simulink model "ofdm_txrx_supermimo_coded"  (Simulink 7.1)
settings: Solver=VariableStepDiscrete  SolverMode=SingleTasking  StartTime=0.0  StopTime=2*simOnly_simLength  RelTol=1e-3
PostLoadFcn:
  | ofdm_rx_supermimo_init
InitFcn:
  | ofdm_rx_supermimo_init
StartFcn:
  | ofdm_rx_supermimo_init
values from neighbouring files (symbols this model uses):
  Action0_Config = 0* 31*2^18 + 0*2^24 + PHY_AUTORESPONSE_REQ_GOODHDR + PHY_AUTORESPONSE_REQ_GOODPKT + PHY_AUTORESPONSE_REQ_FLAGA    [ofdm_tx_supermimo_init.m]
  Action1_Config = 0* 62*2^18 + PHY_AUTORESPONSE_REQ_GOODHDR + PHY_AUTORESPONSE_REQ_GOODPKT    [ofdm_tx_supermimo_init.m]
  Action2_Config = 0    [ofdm_tx_supermimo_init.m]
  Action3_Config = 0    [ofdm_tx_supermimo_init.m]
  Action4_Config = 0    [ofdm_tx_supermimo_init.m]
  Action5_Config = 0    [ofdm_tx_supermimo_init.m]
  ActionID_AFTransmit = 31    [ofdm_tx_supermimo_init.m]
  ActionID_Disabled = 0    [ofdm_tx_supermimo_init.m]
  ActionID_SetFlagA = 62    [ofdm_tx_supermimo_init.m]
  ActionID_SetFlagB = 61    [ofdm_tx_supermimo_init.m]
  BER_RAM_init_size = 2048    [ofdm_tx_supermimo_init.m]
  channel_h = [1 0; 1 0] * 0.3    [ofdm_tx_supermimo_init.m]
  CPLength = 16    [ofdm_tx_supermimo_init.m]
  FEC_Config = fec_coding_en*1 + fec_soft_dec*2 + fec_qpsk_scl*2^4 + fec_16qam_scl*2^8    (= 4195)    [ofdm_tx_supermimo_init.m]
  longCorr_coef_i = [-4*real(LTS) 0]    [ofdm_rx_supermimo_init.m]
  longCorr_coef_q = [4*imag(LTS) 0]    [ofdm_rx_supermimo_init.m]
  Match0_Config = 0    [ofdm_tx_supermimo_init.m]
  Match1_Config = 0    [ofdm_tx_supermimo_init.m]
  Match2_Config = 0    [ofdm_tx_supermimo_init.m]
  Match3_Config = 0    [ofdm_tx_supermimo_init.m]
  Match4_Config = 0    [ofdm_tx_supermimo_init.m]
  Match5_Config = 0    [ofdm_tx_supermimo_init.m]
  Match6_Config = 0    [ofdm_tx_supermimo_init.m]
  Match7_Config = 0    [ofdm_tx_supermimo_init.m]
  max_CP_length = 16    [ofdm_tx_supermimo_init.m]
  max_num_baseRateSymbols = 31    [ofdm_tx_supermimo_init.m]
  max_num_subcarriers = 64    [ofdm_tx_supermimo_init.m]
  max_num_trainingSymbols = 15    [ofdm_tx_supermimo_init.m]
  max_numBytes = 16384    [ofdm_tx_supermimo_init.m]
  max_OFDM_symbols = 1023    [ofdm_tx_supermimo_init.m]
  modConstellation_qam16 = .75*[1 3 -1 -3]./3    [ofdm_tx_supermimo_init.m]
  modConstellation_qam64 = 0.875*[3 1 5 7 -3 -1 -5 -7]./7    [ofdm_tx_supermimo_init.m]
  modConstellation_qpsk = [1 -1]./sqrt(2)    [ofdm_tx_supermimo_init.m]
  numBaseRateSymbols = numBaseRateSymbols *2    [ofdm_tx_supermimo_init.m]
  numSubcarriers = 64    [ofdm_tx_supermimo_init.m]
  numTrainingSymbols = 2    [ofdm_tx_supermimo_init.m]
  pilot_indicies = 7 + ( (64-21) * 2^8) + ( (64-7) * 2^16) + (21 * 2^24)    (= 356068103)    [ofdm_tx_supermimo_init.m]
  pilotValues = hex2dec('A57D') + (2^16 * hex2dec('5A82'))    [ofdm_tx_supermimo_init.m]
  pktByteNums = numHeaderBytes +  				byteIndex_numPayloadBytes(1)*2^8 +  				byteIndex_numPayloadBytes(2)*2^16 +  				byteIndex_simpleDynModMasks * 2^24    [ofdm_rx_supermimo_init.m]
  PktDet_Delay = 58+32 +      Symbol_Timing_Offset*2^7 +      0*2^16 +      (117* 2^24)    (= 1962935642)    [ofdm_rx_supermimo_init.m]
  pktTiming_count_payloadStart = pktTiming_count_lsStart + pktTiming_ls_num*pktTiming_ls_length    [ofdm_rx_supermimo_init.m]
  preamble = preamble_scale*[shortsyms_10 longsyms_2]    [ofdm_tx_supermimo_init.m]
  preamble_ROM = preamble_I_ROM + preamble_Q_ROM*2^16    [ofdm_tx_supermimo_init.m]
  RAM_init_size = max_numBytes    (= 16384)    [ofdm_tx_supermimo_init.m]
  reg_AF_Tx_Blanking = (320+80) + ( (320+80+79)*2^16)    (= 31392144)    [ofdm_rx_supermimo_init.m]
  reg_AF_Tx_Scaling = 2944    [ofdm_rx_supermimo_init.m]
  reg_Interrupt_PktBuf_Control = reg_InterruptControl +  	1 * 2^16 +  	0 * 2^24    (= 65536)    [ofdm_rx_supermimo_init.m]
  reg_PilotCalcParams = (4 * 2^16) + (minPilotChanMag)    (= 262184.96)    [ofdm_rx_supermimo_init.m]
  regRx_chanEst_minMag = ((0.5)*2^15) + ((0.5 * 2^15)*2^16)    (= 1073758208)    [ofdm_rx_supermimo_init.m]
  regRx_coarseCalcCorrection = round((1.3e-3 - 1.2982e-3)*2^32)    [ofdm_rx_supermimo_init.m]
  regRx_pilotCalcCorrection = round(1.00115 * 2^31)    [ofdm_rx_supermimo_init.m]
  regRx_preCFOoptions = 0 * 2^0 +  	1 * 2^1 +  	0    (= 2)    [ofdm_rx_supermimo_init.m]
  rx_controlBits = 1 * 2  		 + 1 * 4  		 + 0 * 8  		 + 1 * 16 * rx_SISO_Mode  		 + 1 * 32  		 + 1 * 64  		 + 1 * 128  		 + 1 * 256  		 + 1 * 512  		 + 1 * 1024  		 + 1 * 4096  		 + 0 * 16384  		 + 0 * 32768  		 + 1 * 65536  		 + 0 * 131072  		 + 0 * 262144  		 + 1 * 524288  		 + 1 * 1048576 * tx_Alamouti_Mode  		 + 0 * 2097152           + 1 * 33554432  		 + 0 * 4194304  		 + 0 * 8388608  		 + 0 * 16777216  		 + 1 *  ...(+49 chars)    [ofdm_rx_supermimo_init.m]
  Rx_PktDet_LongCorr_Params = LongCorrCounterLoad + 0*2^8 + 0*2^16 + 255*2^24    (= 4278190325)    [ofdm_rx_supermimo_init.m]
  Rx_PktDet_LongCorrThresholds = 8000    [ofdm_rx_supermimo_init.m]
  rx_postEq_scaling = round(rxScaling*2^11) * (1 + 2^16)    [ofdm_rx_supermimo_init.m]
  RxDataScrambling_Seq = [40 198 78 63 82 173 102 245 48 111 172 115 147 230 216 93 72 65 62 2 205 242 122 90 128 83 105 97 73 10 5 252]    [ofdm_tx_supermimo_init.m]
  RxReg_FixedPktLen = 0    [ofdm_rx_supermimo_init.m]
  rxReg_pktDetCorr = 2^00 * (floor(0.7*128)) +  	2^08 * (300) +  	0    [ofdm_rx_supermimo_init.m]
  shortSymbol_time = shortSymbol_time(1:16).'    [ofdm_tx_supermimo_init.m]
  simOnly_numSamples = length(preamble)+( (numSubcarriers+CPLength)*(numTrainingSymbols + numBaseRateSymbols + numFullRateSymbols/2) )    [ofdm_tx_supermimo_init.m]
  train = [train train]    [ofdm_tx_supermimo_init.m]
  tx_controlBits = (antB_preambleShift * 2^4) + (2^1 * tx_Alamouti_Mode) +  	(2^0 * tx_SISO_Mode) + 4*0 + 8*1 + 0*256 + 512*0 + 1024*0 +  	0*hex2dec('1000') + 0*hex2dec('2000') + 0*hex2dec('4000') +  	1*hex2dec('8000') + 1*hex2dec('10000') + hex2dec('20000') +  	0*hex2dec('100000')    [ofdm_tx_supermimo_init.m]
  tx_delays = (50*2^0) + (0*2^8) + (30*2^12)    (= 122930)    [ofdm_tx_supermimo_init.m]
  tx_scaling = (3072) + (12288 * 2^16)    (= 805309440)    [ofdm_tx_supermimo_init.m]
  TxDataScrambling_Seq = [40 198 78 63 82 173 102 245 48 111 172 115 147 230 216 93 72 65 62 2 205 242 122 90 128 83 105 97 73 10 5 252]    [ofdm_tx_supermimo_init.m]
  txReg_symbolCounts = (2^8 *numBaseRateSymbols ) + numTrainingSymbols    [ofdm_tx_supermimo_init.m]
  TxRx_FFTScaling = (3 * 2^0) +  	(2 * 2^2) +  	(1 * 2^4) +  	(1 * 2^6) +  	(1 * 2^8) +  	(0 * 2^10)    (= 347)    [ofdm_tx_supermimo_init.m]
undefined symbols (not defined in the file or in its folder): AntA, AntB, BaseRate, Down, gcbh, numBuffs, numBytes, Up, xlBlockLoadCallback, xlGetSimulinkPeriod, xlipgetpartsetting
libraries: xbsIndex_r4 x6000, simulink x4, dspsigops x4, commchan3 x2, dspsrcs4 x2, commrflib2 x2
(part 5 of 63)

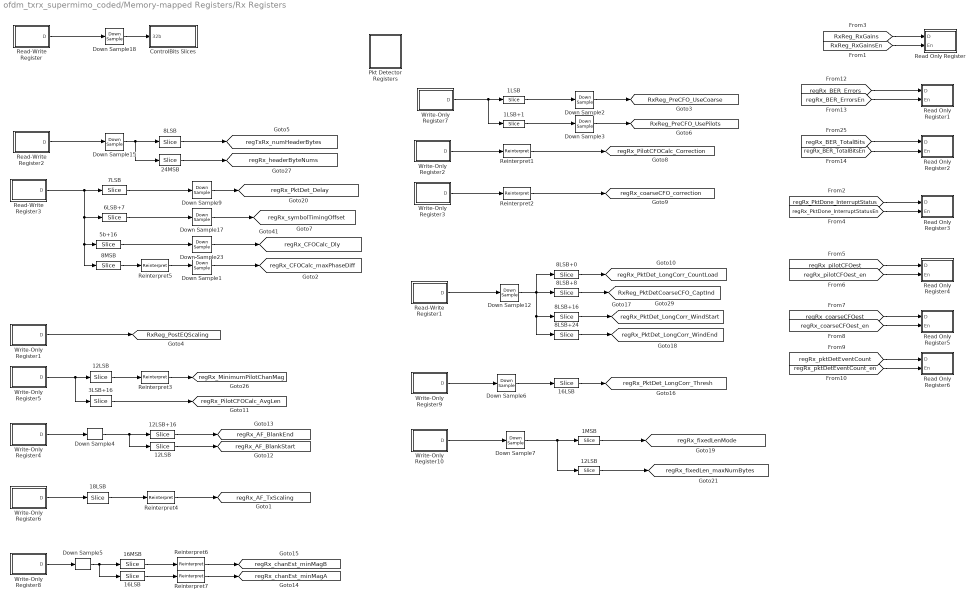
== system ofdm_txrx_supermimo_coded/Memory-mapped Registers/Rx Registers ==
  12LSB : xbsIndex_r4/Slice  nbits=12  boolean_output=off  mode=Lower Bit Location + Width  bit1=0  base1=MSB of Input  bit0=0  base0=LSB of Input  dbl_ovrd=off  block_type=slice
      settings: infoedit="Extracts a given range of bits from each input sample and presents it at the output.  The output type is ordinarily unsigned with binary point at zero, but can be Boolean when the slice is one bit wide.<P><P>Hardware notes: In hardware this block costs nothing."  block_version=VER_STRING_GOES_HERE
  "12LSB " : xbsIndex_r4/Slice  nbits=12  boolean_output=off  mode=Lower Bit Location + Width  bit1=0  base1=MSB of Input  bit0=0  base0=LSB of Input  dbl_ovrd=off  block_type=slice
      settings: infoedit="Extracts a given range of bits from each input sample and presents it at the output.  The output type is ordinarily unsigned with binary point at zero, but can be Boolean when the slice is one bit wide.<P><P>Hardware notes: In hardware this block costs nothing."  block_version=VER_STRING_GOES_HERE
  "12LSB  " : xbsIndex_r4/Slice  nbits=12  boolean_output=off  mode=Lower Bit Location + Width  bit1=0  base1=MSB of Input  bit0=0  base0=LSB of Input  dbl_ovrd=off  block_type=slice
      settings: infoedit="Extracts a given range of bits from each input sample and presents it at the output.  The output type is ordinarily unsigned with binary point at zero, but can be Boolean when the slice is one bit wide.<P><P>Hardware notes: In hardware this block costs nothing."  block_version=VER_STRING_GOES_HERE
  12LSB+16 : xbsIndex_r4/Slice  nbits=12  boolean_output=off  mode=Lower Bit Location + Width  bit1=0  base1=MSB of Input  bit0=16  base0=LSB of Input  dbl_ovrd=off  block_type=slice
      settings: infoedit="Extracts a given range of bits from each input sample and presents it at the output.  The output type is ordinarily unsigned with binary point at zero, but can be Boolean when the slice is one bit wide.<P><P>Hardware notes: In hardware this block costs nothing."  block_version=VER_STRING_GOES_HERE
  16LSB : xbsIndex_r4/Slice  nbits=16  boolean_output=off  mode=Lower Bit Location + Width  bit1=0  base1=MSB of Input  bit0=0  base0=LSB of Input  dbl_ovrd=off  block_type=slice
      settings: infoedit="Extracts a given range of bits from each input sample and presents it at the output.  The output type is ordinarily unsigned with binary point at zero, but can be Boolean when the slice is one bit wide.<P><P>Hardware notes: In hardware this block costs nothing."  block_version=VER_STRING_GOES_HERE
  "16LSB " : xbsIndex_r4/Slice  nbits=16  boolean_output=off  mode=Lower Bit Location + Width  bit1=0  base1=MSB of Input  bit0=0  base0=LSB of Input  dbl_ovrd=off  block_type=slice
      settings: infoedit="Extracts a given range of bits from each input sample and presents it at the output.  The output type is ordinarily unsigned with binary point at zero, but can be Boolean when the slice is one bit wide.<P><P>Hardware notes: In hardware this block costs nothing."  block_version=VER_STRING_GOES_HERE
  "16MSB  " : xbsIndex_r4/Slice  nbits=16  boolean_output=off  mode=Lower Bit Location + Width  bit1=0  base1=MSB of Input  bit0=16  base0=LSB of Input  dbl_ovrd=off  block_type=slice
      settings: infoedit="Extracts a given range of bits from each input sample and presents it at the output.  The output type is ordinarily unsigned with binary point at zero, but can be Boolean when the slice is one bit wide.<P><P>Hardware notes: In hardware this block costs nothing."  block_version=VER_STRING_GOES_HERE
  18LSB : xbsIndex_r4/Slice  nbits=18  boolean_output=off  mode=Lower Bit Location + Width  bit1=0  base1=MSB of Input  bit0=0  base0=LSB of Input  dbl_ovrd=off  block_type=slice
      settings: infoedit="Extracts a given range of bits from each input sample and presents it at the output.  The output type is ordinarily unsigned with binary point at zero, but can be Boolean when the slice is one bit wide.<P><P>Hardware notes: In hardware this block costs nothing."  block_version=VER_STRING_GOES_HERE
  "1LSB " : xbsIndex_r4/Slice  nbits=1  boolean_output=off  mode=Lower Bit Location + Width  bit1=0  base1=MSB of Input  bit0=0  base0=LSB of Input  dbl_ovrd=off  block_type=slice
      settings: infoedit="Extracts a given range of bits from each input sample and presents it at the output.  The output type is ordinarily unsigned with binary point at zero, but can be Boolean when the slice is one bit wide.<P><P>Hardware notes: In hardware this block costs nothing."  block_version=VER_STRING_GOES_HERE
  "1LSB+1 " : xbsIndex_r4/Slice  nbits=1  boolean_output=off  mode=Lower Bit Location + Width  bit1=0  base1=MSB of Input  bit0=1  base0=LSB of Input  dbl_ovrd=off  block_type=slice
      settings: infoedit="Extracts a given range of bits from each input sample and presents it at the output.  The output type is ordinarily unsigned with binary point at zero, but can be Boolean when the slice is one bit wide.<P><P>Hardware notes: In hardware this block costs nothing."  block_version=VER_STRING_GOES_HERE
  1MSB : xbsIndex_r4/Slice  nbits=1  boolean_output=off  mode=Upper Bit Location + Width  bit1=0  base1=MSB of Input  bit0=0  base0=LSB of Input  dbl_ovrd=off  block_type=slice
      settings: infoedit="Extracts a given range of bits from each input sample and presents it at the output.  The output type is ordinarily unsigned with binary point at zero, but can be Boolean when the slice is one bit wide.<P><P>Hardware notes: In hardware this block costs nothing."  block_version=VER_STRING_GOES_HERE
  24MSB : xbsIndex_r4/Slice  nbits=24  boolean_output=off  mode=Lower Bit Location + Width  bit1=0  base1=MSB of Input  bit0=8  base0=LSB of Input  dbl_ovrd=off  block_type=slice
      settings: infoedit="Extracts a given range of bits from each input sample and presents it at the output.  The output type is ordinarily unsigned with binary point at zero, but can be Boolean when the slice is one bit wide.<P><P>Hardware notes: In hardware this block costs nothing."  block_version=VER_STRING_GOES_HERE
  3LSB+16 : xbsIndex_r4/Slice  nbits=3  boolean_output=off  mode=Lower Bit Location + Width  bit1=0  base1=MSB of Input  bit0=16  base0=LSB of Input  dbl_ovrd=off  block_type=slice
      settings: infoedit="Extracts a given range of bits from each input sample and presents it at the output.  The output type is ordinarily unsigned with binary point at zero, but can be Boolean when the slice is one bit wide.<P><P>Hardware notes: In hardware this block costs nothing."  block_version=VER_STRING_GOES_HERE
  5b+16 : xbsIndex_r4/Slice  nbits=5  boolean_output=off  mode=Lower Bit Location + Width  bit1=0  base1=MSB of Input  bit0=16  base0=LSB of Input  dbl_ovrd=off  block_type=slice
      settings: infoedit="Extracts a given range of bits from each input sample and presents it at the output.  The output type is ordinarily unsigned with binary point at zero, but can be Boolean when the slice is one bit wide.<P><P>Hardware notes: In hardware this block costs nothing."  block_version=VER_STRING_GOES_HERE
  6LSB+7 : xbsIndex_r4/Slice  nbits=6  boolean_output=off  mode=Lower Bit Location + Width  bit1=0  base1=MSB of Input  bit0=7  base0=LSB of Input  dbl_ovrd=off  block_type=slice
      settings: infoedit="Extracts a given range of bits from each input sample and presents it at the output.  The output type is ordinarily unsigned with binary point at zero, but can be Boolean when the slice is one bit wide.<P><P>Hardware notes: In hardware this block costs nothing."  block_version=VER_STRING_GOES_HERE
  7LSB : xbsIndex_r4/Slice  nbits=7  boolean_output=off  mode=Lower Bit Location + Width  bit1=0  base1=MSB of Input  bit0=0  base0=LSB of Input  dbl_ovrd=off  block_type=slice
      settings: infoedit="Extracts a given range of bits from each input sample and presents it at the output.  The output type is ordinarily unsigned with binary point at zero, but can be Boolean when the slice is one bit wide.<P><P>Hardware notes: In hardware this block costs nothing."  block_version=VER_STRING_GOES_HERE
  "8LSB " : xbsIndex_r4/Slice  nbits=8  boolean_output=off  mode=Lower Bit Location + Width  bit1=0  base1=MSB of Input  bit0=0  base0=LSB of Input  dbl_ovrd=off  block_type=slice
      settings: infoedit="Extracts a given range of bits from each input sample and presents it at the output.  The output type is ordinarily unsigned with binary point at zero, but can be Boolean when the slice is one bit wide.<P><P>Hardware notes: In hardware this block costs nothing."  block_version=VER_STRING_GOES_HERE
  8LSB+0 : xbsIndex_r4/Slice  nbits=8  boolean_output=off  mode=Lower Bit Location + Width  bit1=0  base1=MSB of Input  bit0=0  base0=LSB of Input  dbl_ovrd=off  block_type=slice
      settings: infoedit="Extracts a given range of bits from each input sample and presents it at the output.  The output type is ordinarily unsigned with binary point at zero, but can be Boolean when the slice is one bit wide.<P><P>Hardware notes: In hardware this block costs nothing."  block_version=VER_STRING_GOES_HERE
  8LSB+16 : xbsIndex_r4/Slice  nbits=8  boolean_output=off  mode=Lower Bit Location + Width  bit1=0  base1=MSB of Input  bit0=16  base0=LSB of Input  dbl_ovrd=off  block_type=slice
      settings: infoedit="Extracts a given range of bits from each input sample and presents it at the output.  The output type is ordinarily unsigned with binary point at zero, but can be Boolean when the slice is one bit wide.<P><P>Hardware notes: In hardware this block costs nothing."  block_version=VER_STRING_GOES_HERE
  8LSB+24 : xbsIndex_r4/Slice  nbits=8  boolean_output=off  mode=Lower Bit Location + Width  bit1=0  base1=MSB of Input  bit0=24  base0=LSB of Input  dbl_ovrd=off  block_type=slice
      settings: infoedit="Extracts a given range of bits from each input sample and presents it at the output.  The output type is ordinarily unsigned with binary point at zero, but can be Boolean when the slice is one bit wide.<P><P>Hardware notes: In hardware this block costs nothing."  block_version=VER_STRING_GOES_HERE
  8LSB+8 : xbsIndex_r4/Slice  nbits=8  boolean_output=off  mode=Lower Bit Location + Width  bit1=0  base1=MSB of Input  bit0=8  base0=LSB of Input  dbl_ovrd=off  block_type=slice
      settings: infoedit="Extracts a given range of bits from each input sample and presents it at the output.  The output type is ordinarily unsigned with binary point at zero, but can be Boolean when the slice is one bit wide.<P><P>Hardware notes: In hardware this block costs nothing."  block_version=VER_STRING_GOES_HERE
  8MSB : xbsIndex_r4/Slice  nbits=8  boolean_output=off  mode=Lower Bit Location + Width  bit1=0  base1=MSB of Input  bit0=24  base0=LSB of Input  dbl_ovrd=off  block_type=slice
      settings: infoedit="Extracts a given range of bits from each input sample and presents it at the output.  The output type is ordinarily unsigned with binary point at zero, but can be Boolean when the slice is one bit wide.<P><P>Hardware notes: In hardware this block costs nothing."  block_version=VER_STRING_GOES_HERE
  ControlBits Slices : Subsystem
  Down Sample{1..4,6,9,12,23} (8 alike) : xbsIndex_r4/Down Sample  sample_ratio=4  sample_phase="Last Value of Frame  (most efficient)"  en=off  latency=1  dbl_ovrd=off  block_type=dsamp
      settings: block_version=VER_STRING_GOES_HERE
  Down Sample{5,7,15,17..18} (5 alike) : xbsIndex_r4/Down Sample  sample_ratio=2  sample_phase="Last Value of Frame  (most efficient)"  en=off  latency=1  dbl_ovrd=off  block_type=dsamp
      settings: block_version=VER_STRING_GOES_HERE
  Pkt Detector Registers : Subsystem
  Read Only Register : Subsystem  AncestorBlock=|  mask{regName='Rx_Gains'}
      | WARP_Blockset/Register Wrappers
      | (Sysgen 10.1+)/Read Only Register
      mask prompts: regName "Register Name"
      mask help:
      | This block is a wrapper for System Generator's To Register shared memory block. This register is designed for use with WARP's sysgen2opb tool.
      |
      | Note: The Regisger Name paramter below must be unique for every instance of Read-Write and Read-Only registers in your system.
      mask init:
      | regBlock = find_system(gcb, 'LookUnderMasks', 'all', 'FollowLinks', 'on',   'block_type', 'toreg');
      | 
      | if(length(regBlock) > 0)
      | 
      |     %Set the shared memory name
      |     set_param(regBlock{1}, 'shared_memory_name', regName);
      | 
      | 	set_param(regBlock{1}, 'explicit_data_type', 'on')
      |     set_param(regBlock{1}, 'arith_type', 'unsigned');
      | 	set_param(regBlock{1}, 'ownership', 'Locally owned and initialized')
      | 
      | 	set_param(regBlock{1}, 'n_bits', '32');
      | 	set_param(regBlock{1}, 'bin_pt', '0');
      | 	set_param(regBlock{1}, 'init', '0');
      | end
      settings: AttributesFormatString=RO Reg: %<regName>
  Read Only Register1 : Subsystem  AncestorBlock=|  mask{regName='Rx_BER_Errors'}
      | WARP_Blockset/Register Wrappers
      | (Sysgen 10.1+)/Read Only Register
      mask prompts: regName "Register Name"
      mask help:
      | This block is a wrapper for System Generator's To Register shared memory block. This register is designed for use with WARP's sysgen2opb tool.
      |
      | Note: The Regisger Name paramter below must be unique for every instance of Read-Write and Read-Only registers in your system.
      mask init: same as ofdm_txrx_supermimo_coded/Memory-mapped Registers/Rx Registers/Read Only Register
      settings: AttributesFormatString=RO Reg: %<regName>
  Read Only Register2 : Subsystem  AncestorBlock=|  mask{regName='Rx_BER_TotalBits'}
      | WARP_Blockset/Register Wrappers
      | (Sysgen 10.1+)/Read Only Register
      mask prompts: regName "Register Name"
      mask help:
      | This block is a wrapper for System Generator's To Register shared memory block. This register is designed for use with WARP's sysgen2opb tool.
      |
      | Note: The Regisger Name paramter below must be unique for every instance of Read-Write and Read-Only registers in your system.
      mask init: same as ofdm_txrx_supermimo_coded/Memory-mapped Registers/Rx Registers/Read Only Register
      settings: AttributesFormatString=RO Reg: %<regName>
  Read Only Register3 : Subsystem  AncestorBlock=|  mask{regName='Rx_pktDone_interruptStatus'}
      | WARP_Blockset/Register Wrappers
      | (Sysgen 10.1+)/Read Only Register
      mask prompts: regName "Register Name"
      mask help:
      | This block is a wrapper for System Generator's To Register shared memory block. This register is designed for use with WARP's sysgen2opb tool.
      |
      | Note: The Regisger Name paramter below must be unique for every instance of Read-Write and Read-Only registers in your system.
      mask init: same as ofdm_txrx_supermimo_coded/Memory-mapped Registers/Rx Registers/Read Only Register
      settings: AttributesFormatString=RO Reg: %<regName>
  Read Only Register4 : Subsystem  AncestorBlock=|  mask{regName='Rx_pilotCFOest'}
      | WARP_Blockset/Register Wrappers
      | (Sysgen 10.1+)/Read Only Register
      mask prompts: regName "Register Name"
      mask help:
      | This block is a wrapper for System Generator's To Register shared memory block. This register is designed for use with WARP's sysgen2opb tool.
      |
      | Note: The Regisger Name paramter below must be unique for every instance of Read-Write and Read-Only registers in your system.
      mask init: same as ofdm_txrx_supermimo_coded/Memory-mapped Registers/Rx Registers/Read Only Register
      settings: AttributesFormatString=RO Reg: %<regName>
  Read Only Register5 : Subsystem  AncestorBlock=|  mask{regName='Rx_coarseCFOest'}
      | WARP_Blockset/Register Wrappers
      | (Sysgen 10.1+)/Read Only Register
      mask prompts: regName "Register Name"
      mask help:
      | This block is a wrapper for System Generator's To Register shared memory block. This register is designed for use with WARP's sysgen2opb tool.
      |
      | Note: The Regisger Name paramter below must be unique for every instance of Read-Write and Read-Only registers in your system.
      mask init: same as ofdm_txrx_supermimo_coded/Memory-mapped Registers/Rx Registers/Read Only Register
      settings: AttributesFormatString=RO Reg: %<regName>
  Read Only Register6 : Subsystem  AncestorBlock=|  mask{regName='Rx_pktDetEventCount'}
      | WARP_Blockset/Register Wrappers
      | (Sysgen 10.1+)/Read Only Register
      mask prompts: regName "Register Name"
      mask help:
      | This block is a wrapper for System Generator's To Register shared memory block. This register is designed for use with WARP's sysgen2opb tool.
      |
      | Note: The Regisger Name paramter below must be unique for every instance of Read-Write and Read-Only registers in your system.
      mask init: same as ofdm_txrx_supermimo_coded/Memory-mapped Registers/Rx Registers/Read Only Register
      settings: AttributesFormatString=RO Reg: %<regName>
  Read-Write Register : Subsystem  AncestorBlock=|  mask{regName='Rx_ControlBits', out_dataType=Unsigned, out_numBits=32, out_binaryPt=0, initValue=rx_controlBits, sampPeriod=1}
      | WARP_Blockset/Register Wrappers
      | (Sysgen 10.1+)/Read-Write Register
      mask prompts: regName "Register Name"; out_dataType "Output Type"; out_numBits "Output Num Bits"; out_binaryPt "Output Binary Point"; initValue "Initial Value"; sampPeriod "Sample Period"
      mask help:
      | This block is a wrapper for two registers from the System Generator Shared Memory blockset:
      | a) A "From Register" block, which provides a write-only register for the host processor
      | b) A "To Register" block, which provides a read-only register for the host processor
      |
      | Note: The Regisger Name paramter below must be unique for every instance of shared-memory registers in your system. System Generator will throw an error if duplicate names are found.
      mask init: same as ofdm_txrx_supermimo_coded/Memory-mapped Registers/AutoReply Registers/Read-Write Register1
      settings: AttributesFormatString=RW Reg: %<regName>
      mask StyleString: edit,popup(Unsigned|Signed  (2's comp)),edit,edit,edit,edit
  Read-Write Register1 : Subsystem  AncestorBlock=|  mask{regName='Rx_PktDet_LongCorr_Params', out_dataType=Unsigned, out_numBits=32, out_binaryPt=0, initValue=Rx_PktDet_LongCorr_Params, sampPeriod=1}
      | WARP_Blockset/Register Wrappers
      | (Sysgen 10.1+)/Read-Write Register
      mask prompts: regName "Register Name"; out_dataType "Output Type"; out_numBits "Output Num Bits"; out_binaryPt "Output Binary Point"; initValue "Initial Value"; sampPeriod "Sample Period"
      mask help:
      | This block is a wrapper for two registers from the System Generator Shared Memory blockset:
      | a) A "From Register" block, which provides a write-only register for the host processor
      | b) A "To Register" block, which provides a read-only register for the host processor
      |
      | Note: The Regisger Name paramter below must be unique for every instance of shared-memory registers in your system. System Generator will throw an error if duplicate names are found.
      mask init: same as ofdm_txrx_supermimo_coded/Memory-mapped Registers/AutoReply Registers/Read-Write Register1
      settings: AttributesFormatString=RW Reg: %<regName>
      mask StyleString: edit,popup(Unsigned|Signed  (2's comp)),edit,edit,edit,edit
  Read-Write Register2 : Subsystem  AncestorBlock=|  mask{regName='Rx_pktByteNums', out_dataType=Unsigned, out_numBits=32, out_binaryPt=0, initValue=pktByteNums, sampPeriod=1}
      | WARP_Blockset/Register Wrappers
      | (Sysgen 10.1+)/Read-Write Register
      mask prompts: regName "Register Name"; out_dataType "Output Type"; out_numBits "Output Num Bits"; out_binaryPt "Output Binary Point"; initValue "Initial Value"; sampPeriod "Sample Period"
      mask help:
      | This block is a wrapper for two registers from the System Generator Shared Memory blockset:
      | a) A "From Register" block, which provides a write-only register for the host processor
      | b) A "To Register" block, which provides a read-only register for the host processor
      |
      | Note: The Regisger Name paramter below must be unique for every instance of shared-memory registers in your system. System Generator will throw an error if duplicate names are found.
      mask init: same as ofdm_txrx_supermimo_coded/Memory-mapped Registers/AutoReply Registers/Read-Write Register1
      settings: AttributesFormatString=RW Reg: %<regName>
      mask StyleString: edit,popup(Unsigned|Signed  (2's comp)),edit,edit,edit,edit
  Read-Write Register3 : Subsystem  AncestorBlock=|  mask{regName='Rx_PktDet_Delay', out_dataType=Unsigned, out_numBits=32, out_binaryPt=0, initValue=PktDet_Delay, sampPeriod=1}
      | WARP_Blockset/Register Wrappers
      | (Sysgen 10.1+)/Read-Write Register
      mask prompts: regName "Register Name"; out_dataType "Output Type"; out_numBits "Output Num Bits"; out_binaryPt "Output Binary Point"; initValue "Initial Value"; sampPeriod "Sample Period"
      mask help:
      | This block is a wrapper for two registers from the System Generator Shared Memory blockset:
      | a) A "From Register" block, which provides a write-only register for the host processor
      | b) A "To Register" block, which provides a read-only register for the host processor
      |
      | Note: The Regisger Name paramter below must be unique for every instance of shared-memory registers in your system. System Generator will throw an error if duplicate names are found.
      mask init: same as ofdm_txrx_supermimo_coded/Memory-mapped Registers/AutoReply Registers/Read-Write Register1
      settings: AttributesFormatString=RW Reg: %<regName>
      mask StyleString: edit,popup(Unsigned|Signed  (2's comp)),edit,edit,edit,edit
  Reinterpret1 : xbsIndex_r4/Reinterpret  force_arith_type=on  arith_type=Unsigned  force_bin_pt=on  bin_pt=31  block_type=reinterpret
      settings: infoedit="Changes signal type without altering the binary representation.   You can changed the signal between signed and unsigned, and relocate the binary point.<P><P>Hardware notes: In hardware this block costs nothing.<P><P>Example:  Suppose the input is 6 bits wide, signed, with 2 fractional bits, and the output is forced to unsigned with 0 fractional bits.  Then an input of -2.0 (1110.00 in binary 2's complement) becomes an output of 56 (111000 in binary)."  block_version=10.1
  Reinterpret2 : xbsIndex_r4/Reinterpret  force_arith_type=on  arith_type="Signed  (2's comp)"  force_bin_pt=on  bin_pt=32  block_type=reinterpret
      settings: infoedit="Changes signal type without altering the binary representation.   You can changed the signal between signed and unsigned, and relocate the binary point.<P><P>Hardware notes: In hardware this block costs nothing.<P><P>Example:  Suppose the input is 6 bits wide, signed, with 2 fractional bits, and the output is forced to unsigned with 0 fractional bits.  Then an input of -2.0 (1110.00 in binary 2's complement) becomes an output of 56 (111000 in binary)."  block_version=10.1
  Reinterpret3 : xbsIndex_r4/Reinterpret  force_arith_type=on  arith_type=Unsigned  force_bin_pt=on  bin_pt=12  block_type=reinterpret
      settings: infoedit="Changes signal type without altering the binary representation.   You can changed the signal between signed and unsigned, and relocate the binary point.<P><P>Hardware notes: In hardware this block costs nothing.<P><P>Example:  Suppose the input is 6 bits wide, signed, with 2 fractional bits, and the output is forced to unsigned with 0 fractional bits.  Then an input of -2.0 (1110.00 in binary 2's complement) becomes an output of 56 (111000 in binary)."  block_version=10.1
  Reinterpret4 : xbsIndex_r4/Reinterpret  force_arith_type=on  arith_type=Unsigned  force_bin_pt=on  bin_pt=12  block_type=reinterpret
      settings: infoedit="Changes signal type without altering the binary representation.   You can changed the signal between signed and unsigned, and relocate the binary point.<P><P>Hardware notes: In hardware this block costs nothing.<P><P>Example:  Suppose the input is 6 bits wide, signed, with 2 fractional bits, and the output is forced to unsigned with 0 fractional bits.  Then an input of -2.0 (1110.00 in binary 2's complement) becomes an output of 56 (111000 in binary)."  block_version=10.1
  Reinterpret5 : xbsIndex_r4/Reinterpret  force_arith_type=on  arith_type=Unsigned  force_bin_pt=on  bin_pt=8  block_type=reinterpret
      settings: infoedit="Changes signal type without altering the binary representation.   You can changed the signal between signed and unsigned, and relocate the binary point.<P><P>Hardware notes: In hardware this block costs nothing.<P><P>Example:  Suppose the input is 6 bits wide, signed, with 2 fractional bits, and the output is forced to unsigned with 0 fractional bits.  Then an input of -2.0 (1110.00 in binary 2's complement) becomes an output of 56 (111000 in binary)."  block_version=10.1
  Reinterpret6 : xbsIndex_r4/Reinterpret  force_arith_type=on  arith_type=Unsigned  force_bin_pt=on  bin_pt=15  block_type=reinterpret
      settings: infoedit="Changes signal type without altering the binary representation.   You can changed the signal between signed and unsigned, and relocate the binary point.<P><P>Hardware notes: In hardware this block costs nothing.<P><P>Example:  Suppose the input is 6 bits wide, signed, with 2 fractional bits, and the output is forced to unsigned with 0 fractional bits.  Then an input of -2.0 (1110.00 in binary 2's complement) becomes an output of 56 (111000 in binary)."  block_version=10.1
  Reinterpret7 : xbsIndex_r4/Reinterpret  force_arith_type=on  arith_type=Unsigned  force_bin_pt=on  bin_pt=15  block_type=reinterpret
      settings: infoedit="Changes signal type without altering the binary representation.   You can changed the signal between signed and unsigned, and relocate the binary point.<P><P>Hardware notes: In hardware this block costs nothing.<P><P>Example:  Suppose the input is 6 bits wide, signed, with 2 fractional bits, and the output is forced to unsigned with 0 fractional bits.  Then an input of -2.0 (1110.00 in binary 2's complement) becomes an output of 56 (111000 in binary)."  block_version=10.1
  Write-Only Register1 : Subsystem  AncestorBlock=|  mask{regName='Rx_Constellation_Scaling', out_dataType=Unsigned, out_numBits=32, out_binaryPt=0, initValue=rx_postEq_scaling, sampPeriod=1}
      | WARP_Blockset/Register Wrappers
      | (Sysgen 10.1+)/Write-Only Register
      mask prompts: regName "Register Name"; out_dataType "Output Type"; out_numBits "Output Num Bits"; out_binaryPt "Output Binary Point"; initValue "Initial Value"; sampPeriod "Sample Period"
      mask help:
      | This block is a wrapper for System Generator's "From Register" shared memory block.
      |
      | Note: The Regisger Name paramter below must be unique for every instance of shared-memory registers in your system. System Generator will throw an error if duplicate names are found.
      mask init:
      | regBlock = find_system(gcb, 'LookUnderMasks', 'all', 'FollowLinks', 'on', 'block_type', 'fromreg');
      | 
      | if(length(regBlock)>0)
      | 
      | 	%Set the shared memory name
      | 
      |     set_param(regBlock{1}, 'shared_memory_name', ['''' strcat(regName(2:end-1), '_w') '''']);
      | 
      | 	set_param(regBlock{1}, 'arith_type', sprintf('%s', out_dataType));
      | 	set_param(regBlock{1}, 'ownership', 'Locally owned and initialized')
      | 
      | 	set_param(regBlock{1}, 'n_bits', num2str(out_numBits));
      | 	set_param(regBlock{1}, 'bin_pt', num2str(out_binaryPt));
      | 	set_param(regBlock{1}, 'init', num2str(initValue));
      | end
      settings: AttributesFormatString=RW Reg: %<regName>
      mask StyleString: edit,popup(Unsigned|Signed  (2's comp)),edit,edit,edit,edit
  Write-Only Register10 : Subsystem  AncestorBlock=|  mask{regName='Rx_FixedPktLen', out_dataType=Unsigned, out_numBits=32, out_binaryPt=0, initValue=RxReg_FixedPktLen, sampPeriod=1}
      | WARP_Blockset/Register Wrappers
      | (Sysgen 10.1+)/Write-Only Register
      mask prompts: regName "Register Name"; out_dataType "Output Type"; out_numBits "Output Num Bits"; out_binaryPt "Output Binary Point"; initValue "Initial Value"; sampPeriod "Sample Period"
      mask help:
      | This block is a wrapper for System Generator's "From Register" shared memory block.
      |
      | Note: The Regisger Name paramter below must be unique for every instance of shared-memory registers in your system. System Generator will throw an error if duplicate names are found.
      mask init: same as ofdm_txrx_supermimo_coded/Memory-mapped Registers/Rx Registers/Write-Only Register1
      settings: AttributesFormatString=RW Reg: %<regName>
      mask StyleString: edit,popup(Unsigned|Signed  (2's comp)),edit,edit,edit,edit
  Write-Only Register2 : Subsystem  AncestorBlock=|  mask{regName='Rx_PreCFO_PilotCalcCorrection', out_dataType=Unsigned, out_numBits=32, out_binaryPt=0, initValue=regRx_pilotCalcCorrection, sampPeriod=1}
      | WARP_Blockset/Register Wrappers
      | (Sysgen 10.1+)/Write-Only Register
      mask prompts: regName "Register Name"; out_dataType "Output Type"; out_numBits "Output Num Bits"; out_binaryPt "Output Binary Point"; initValue "Initial Value"; sampPeriod "Sample Period"
      mask help:
      | This block is a wrapper for System Generator's "From Register" shared memory block.
      |
      | Note: The Regisger Name paramter below must be unique for every instance of shared-memory registers in your system. System Generator will throw an error if duplicate names are found.
      mask init: same as ofdm_txrx_supermimo_coded/Memory-mapped Registers/Rx Registers/Write-Only Register1
      settings: AttributesFormatString=RW Reg: %<regName>
      mask StyleString: edit,popup(Unsigned|Signed  (2's comp)),edit,edit,edit,edit
  Write-Only Register3 : Subsystem  AncestorBlock=|  mask{regName='Rx_coarseCFO_correction', out_dataType=Unsigned, out_numBits=32, out_binaryPt=0, initValue=regRx_coarseCalcCorrection, sampPeriod=1}
      | WARP_Blockset/Register Wrappers
      | (Sysgen 10.1+)/Write-Only Register
      mask prompts: regName "Register Name"; out_dataType "Output Type"; out_numBits "Output Num Bits"; out_binaryPt "Output Binary Point"; initValue "Initial Value"; sampPeriod "Sample Period"
      mask help:
      | This block is a wrapper for System Generator's "From Register" shared memory block.
      |
      | Note: The Regisger Name paramter below must be unique for every instance of shared-memory registers in your system. System Generator will throw an error if duplicate names are found.
      mask init: same as ofdm_txrx_supermimo_coded/Memory-mapped Registers/Rx Registers/Write-Only Register1
      settings: AttributesFormatString=RW Reg: %<regName>
      mask StyleString: edit,popup(Unsigned|Signed  (2's comp)),edit,edit,edit,edit
  Write-Only Register4 : Subsystem  AncestorBlock=|  mask{regName='Rx_AF_Blanking', out_dataType=Unsigned, out_numBits=32, out_binaryPt=0, initValue=reg_AF_Tx_Blanking, sampPeriod=1}
      | WARP_Blockset/Register Wrappers
      | (Sysgen 10.1+)/Write-Only Register
      mask prompts: regName "Register Name"; out_dataType "Output Type"; out_numBits "Output Num Bits"; out_binaryPt "Output Binary Point"; initValue "Initial Value"; sampPeriod "Sample Period"
      mask help:
      | This block is a wrapper for System Generator's "From Register" shared memory block.
      |
      | Note: The Regisger Name paramter below must be unique for every instance of shared-memory registers in your system. System Generator will throw an error if duplicate names are found.
      mask init: same as ofdm_txrx_supermimo_coded/Memory-mapped Registers/Rx Registers/Write-Only Register1
      settings: AttributesFormatString=RW Reg: %<regName>
      mask StyleString: edit,popup(Unsigned|Signed  (2's comp)),edit,edit,edit,edit
  Write-Only Register5 : Subsystem  AncestorBlock=|  mask{regName='Rx_PilotCalcParams', out_dataType=Unsigned, out_numBits=32, out_binaryPt=0, initValue=reg_PilotCalcParams, sampPeriod=1}
      | WARP_Blockset/Register Wrappers
      | (Sysgen 10.1+)/Write-Only Register
      mask prompts: regName "Register Name"; out_dataType "Output Type"; out_numBits "Output Num Bits"; out_binaryPt "Output Binary Point"; initValue "Initial Value"; sampPeriod "Sample Period"
      mask help:
      | This block is a wrapper for System Generator's "From Register" shared memory block.
      |
      | Note: The Regisger Name paramter below must be unique for every instance of shared-memory registers in your system. System Generator will throw an error if duplicate names are found.
      mask init: same as ofdm_txrx_supermimo_coded/Memory-mapped Registers/Rx Registers/Write-Only Register1
      settings: AttributesFormatString=RW Reg: %<regName>
      mask StyleString: edit,popup(Unsigned|Signed  (2's comp)),edit,edit,edit,edit
  Write-Only Register6 : Subsystem  AncestorBlock=|  mask{regName='Rx_AF_TxScaling', out_dataType=Unsigned, out_numBits=32, out_binaryPt=0, initValue=reg_AF_Tx_Scaling, sampPeriod=1}
      | WARP_Blockset/Register Wrappers
      | (Sysgen 10.1+)/Write-Only Register
      mask prompts: regName "Register Name"; out_dataType "Output Type"; out_numBits "Output Num Bits"; out_binaryPt "Output Binary Point"; initValue "Initial Value"; sampPeriod "Sample Period"
      mask help:
      | This block is a wrapper for System Generator's "From Register" shared memory block.
      |
      | Note: The Regisger Name paramter below must be unique for every instance of shared-memory registers in your system. System Generator will throw an error if duplicate names are found.
      mask init: same as ofdm_txrx_supermimo_coded/Memory-mapped Registers/Rx Registers/Write-Only Register1
      settings: AttributesFormatString=RW Reg: %<regName>
      mask StyleString: edit,popup(Unsigned|Signed  (2's comp)),edit,edit,edit,edit
  Write-Only Register7 : Subsystem  AncestorBlock=|  mask{regName='Rx_PreCFO_Options', out_dataType=Unsigned, out_numBits=32, out_binaryPt=0, initValue=regRx_preCFOoptions, sampPeriod=1}
      | WARP_Blockset/Register Wrappers
      | (Sysgen 10.1+)/Write-Only Register
      mask prompts: regName "Register Name"; out_dataType "Output Type"; out_numBits "Output Num Bits"; out_binaryPt "Output Binary Point"; initValue "Initial Value"; sampPeriod "Sample Period"
      mask help:
      | This block is a wrapper for System Generator's "From Register" shared memory block.
      |
      | Note: The Regisger Name paramter below must be unique for every instance of shared-memory registers in your system. System Generator will throw an error if duplicate names are found.
      mask init: same as ofdm_txrx_supermimo_coded/Memory-mapped Registers/Rx Registers/Write-Only Register1
      settings: AttributesFormatString=RW Reg: %<regName>
      mask StyleString: edit,popup(Unsigned|Signed  (2's comp)),edit,edit,edit,edit
  Write-Only Register8 : Subsystem  AncestorBlock=|  mask{regName='Rx_ChanEst_MinMag', out_dataType=Unsigned, out_numBits=32, out_binaryPt=0, initValue=regRx_chanEst_minMag, sampPeriod=1}
      | WARP_Blockset/Register Wrappers
      | (Sysgen 10.1+)/Write-Only Register
      mask prompts: regName "Register Name"; out_dataType "Output Type"; out_numBits "Output Num Bits"; out_binaryPt "Output Binary Point"; initValue "Initial Value"; sampPeriod "Sample Period"
      mask help:
      | This block is a wrapper for System Generator's "From Register" shared memory block.
      |
      | Note: The Regisger Name paramter below must be unique for every instance of shared-memory registers in your system. System Generator will throw an error if duplicate names are found.
      mask init: same as ofdm_txrx_supermimo_coded/Memory-mapped Registers/Rx Registers/Write-Only Register1
      settings: AttributesFormatString=RW Reg: %<regName>
      mask StyleString: edit,popup(Unsigned|Signed  (2's comp)),edit,edit,edit,edit
  Write-Only Register9 : Subsystem  AncestorBlock=|  mask{regName='Rx_PktDet_LongCorr_Thresholds', out_dataType=Unsigned, out_numBits=32, out_binaryPt=0, initValue=Rx_PktDet_LongCorrThresholds, sampPeriod=1}
      | WARP_Blockset/Register Wrappers
      | (Sysgen 10.1+)/Write-Only Register
      mask prompts: regName "Register Name"; out_dataType "Output Type"; out_numBits "Output Num Bits"; out_binaryPt "Output Binary Point"; initValue "Initial Value"; sampPeriod "Sample Period"
      mask help:
      | This block is a wrapper for System Generator's "From Register" shared memory block.
      |
      | Note: The Regisger Name paramter below must be unique for every instance of shared-memory registers in your system. System Generator will throw an error if duplicate names are found.
      mask init: same as ofdm_txrx_supermimo_coded/Memory-mapped Registers/Rx Registers/Write-Only Register1
      settings: AttributesFormatString=RW Reg: %<regName>
      mask StyleString: edit,popup(Unsigned|Signed  (2's comp)),edit,edit,edit,edit
  tag scope: RxReg_PktDetCoarseCFO_CaptInd=global, RxReg_PostEQScaling=global, RxReg_PreCFO_UseCoarse=global, RxReg_PreCFO_UsePilots=global, regRx_AF_BlankEnd=global, regRx_AF_BlankStart=global, regRx_AF_TxScaling=global, regRx_CFOCalc_Dly=global, regRx_CFOCalc_maxPhaseDiff=global, regRx_MinimumPilotChanMag=global, regRx_PilotCFOCalc_AvgLen=global, regRx_PilotCFOCalc_Correction=global, regRx_PktDet_Delay=global, regRx_PktDet_LongCorr_CountLoad=global, regRx_PktDet_LongCorr_Thresh=global, regRx_PktDet_LongCorr_WindEnd=global, regRx_PktDet_LongCorr_WindStart=global, regRx_chanEst_minMagA=global, regRx_chanEst_minMagB=global, regRx_coarseCFO_correction=global, regRx_fixedLenMode=global, regRx_fixedLen_maxNumBytes=global, regRx_headerByteNums=global, regRx_symbolTimingOffset=global, regTxRx_numHeaderBytes=global
  wires:
  Read-Write Register/1(D) -> Down Sample18/1
  Read-Write Register2/1(D) -> Down Sample15/1
  Read-Write Register3/1(D) -> 7LSB/1, 6LSB+7/1, 5b+16/1, 8MSB/1
  Down Sample9/1 -> [regRx_PktDet_Delay]
  Down Sample15/1 -> 24MSB/1, "8LSB "/1
  8LSB+8/1 -> [RxReg_PktDetCoarseCFO_CaptInd]
  Read-Write Register1/1(D) -> Down Sample12/1
  Down Sample12/1 -> 8LSB+8/1, 8LSB+0/1, 8LSB+16/1, 8LSB+24/1
  24MSB/1 -> [regRx_headerByteNums]
  7LSB/1 -> Down Sample9/1
  6LSB+7/1 -> Down Sample17/1
  Down Sample17/1 -> [regRx_symbolTimingOffset]
  Down Sample23/1 -> [regRx_CFOCalc_Dly]
  5b+16/1 -> Down Sample23/1
  Down Sample18/1 -> ControlBits Slices/1(32b)
  "8LSB "/1 -> [regTxRx_numHeaderBytes]
  [RxReg_RxGains] -> Read Only Register/1(D)
  [RxReg_RxGainsEn] -> Read Only Register/2(En)
  Write-Only Register1/1(D) -> [RxReg_PostEQScaling]
  [regRx_BER_TotalBitsEn] -> Read Only Register2/2(En)
  [regRx_BER_Errors] -> Read Only Register1/1(D)
  [regRx_BER_TotalBits] -> Read Only Register2/1(D)
  [regRx_BER_ErrorsEn] -> Read Only Register1/2(En)
  [regRx_PktDone_InterruptStatus] -> Read Only Register3/1(D)
  [regRx_PktDone_InterruptStatusEn] -> Read Only Register3/2(En)
  Write-Only Register6/1(D) -> 18LSB/1
  Reinterpret4/1 -> [regRx_AF_TxScaling]
  18LSB/1 -> Reinterpret4/1
  8MSB/1 -> Reinterpret5/1
  Down Sample1/1 -> [regRx_CFOCalc_maxPhaseDiff]
  Reinterpret5/1 -> Down Sample1/1
  "1LSB "/1 -> Down Sample2/1
  Write-Only Register7/1(D) -> "1LSB "/1, "1LSB+1 "/1
  Down Sample2/1 -> [RxReg_PreCFO_UseCoarse]
  Write-Only Register5/1(D) -> 12LSB/1, 3LSB+16/1
  Reinterpret3/1 -> [regRx_MinimumPilotChanMag]
  12LSB/1 -> Reinterpret3/1
  3LSB+16/1 -> [regRx_PilotCFOCalc_AvgLen]
  [regRx_pilotCFOest] -> Read Only Register4/1(D)
  [regRx_pilotCFOest_en] -> Read Only Register4/2(En)
  [regRx_coarseCFOest] -> Read Only Register5/1(D)
  [regRx_coarseCFOest_en] -> Read Only Register5/2(En)
  "1LSB+1 "/1 -> Down Sample3/1
  Down Sample3/1 -> [RxReg_PreCFO_UsePilots]
  [regRx_pktDetEventCount] -> Read Only Register6/1(D)
  [regRx_pktDetEventCount_en] -> Read Only Register6/2(En)
  Reinterpret1/1 -> [regRx_PilotCFOCalc_Correction]
  Write-Only Register2/1(D) -> Reinterpret1/1
  Reinterpret2/1 -> [regRx_coarseCFO_correction]
  Write-Only Register3/1(D) -> Reinterpret2/1
  8LSB+0/1 -> [regRx_PktDet_LongCorr_CountLoad]
  Write-Only Register4/1(D) -> Down Sample4/1
  12LSB+16/1 -> [regRx_AF_BlankEnd]
  "12LSB "/1 -> [regRx_AF_BlankStart]
  Down Sample4/1 -> 12LSB+16/1, "12LSB "/1
  Down Sample5/1 -> 16LSB/1, "16MSB  "/1
  Write-Only Register8/1(D) -> Down Sample5/1
  16LSB/1 -> Reinterpret7/1
  "16MSB  "/1 -> Reinterpret6/1
  Reinterpret6/1 -> [regRx_chanEst_minMagB]
  Reinterpret7/1 -> [regRx_chanEst_minMagA]
  Write-Only Register9/1(D) -> Down Sample6/1
  Down Sample6/1 -> "16LSB "/1
  "16LSB "/1 -> [regRx_PktDet_LongCorr_Thresh]
  8LSB+16/1 -> [regRx_PktDet_LongCorr_WindStart]
  8LSB+24/1 -> [regRx_PktDet_LongCorr_WindEnd]
  Write-Only Register10/1(D) -> Down Sample7/1
  1MSB/1 -> [regRx_fixedLenMode]
  "12LSB  "/1 -> [regRx_fixedLen_maxNumBytes]
  Down Sample7/1 -> 1MSB/1, "12LSB  "/1

== system ofdm_txrx_supermimo_coded/Memory-mapped Registers/Static Parameters (might be registers someday) ==
  note: Replace with registers someday
      | to support dynamic transform and
      | cyclic prefix sizes
  Constant : xbsIndex_r4/Constant  arith_type=Unsigned  const=numSubcarriers  n_bits=ceil(log2(max_num_subcarriers)+1)  bin_pt=0  explicit_period=on  period=2  equ=P=C  opselect=C  inp2=PCIN>>17  opr=+  inp1=P  carry=CIN  dbl_ovrd=off  block_type=constant
      settings: block_version=VER_STRING_GOES_HERE
  Constant1 : xbsIndex_r4/Constant  arith_type=Unsigned  const=numSubcarriers+CPLength-1  n_bits=ceil(log2(max_num_subcarriers+CPLength)+1)  bin_pt=0  explicit_period=on  period=2  equ=P=C  opselect=C  inp2=PCIN>>17  opr=+  inp1=P  carry=CIN  dbl_ovrd=off  block_type=constant
      settings: block_version=VER_STRING_GOES_HERE
  Constant2 : xbsIndex_r4/Constant  arith_type=Unsigned  const=16  n_bits=5  bin_pt=0  explicit_period=on  period=1  equ=P=C  opselect=C  inp2=PCIN>>17  opr=+  inp1=P  carry=CIN  dbl_ovrd=off  block_type=constant
      settings: block_version=VER_STRING_GOES_HERE
  Down Sample8 : xbsIndex_r4/Down Sample  sample_ratio=2  sample_phase="Last Value of Frame  (most efficient)"  en=off  latency=1  dbl_ovrd=off  block_type=dsamp
      settings: block_version=VER_STRING_GOES_HERE
  tag scope: regRX_CP_Length=global, regRX_numSubcarriers=global, regRX_sampsPerSymMinusOne=global
  wires:
  Constant/1 -> [regRX_numSubcarriers]
  Constant1/1 -> [regRX_sampsPerSymMinusOne]
  Down Sample8/1 -> [regRX_CP_Length]
  Constant2/1 -> Down Sample8/1
